simulink model "MX_Gateway"  (Simulink 9.0)
settings: Solver=FixedStepDiscrete  StartTime=0.0  StopTime=100  FixedStep=0.01  RelTol=1e-3
values from neighbouring files (symbols this model uses):
  ACTIVE = Bobcat.Parameter; CreateDefine( ACTIVE                         ,1                          ,'Define' ,'defines.h' )    [defines.m]
  ACTIVE_MODE_ONE = Bobcat.Parameter; CreateDefine( ACTIVE_MODE_ONE                ,19                         ,'Define' ,'defines.h' )    [defines.m]
  AUTHENTICATED = Bobcat.Parameter; CreateDefine( AUTHENTICATED                  ,1                          ,'Define' ,'defines.h' )    [defines.m]
  AUX_EMEA_CONTROLS = Bobcat.Parameter; CreateDefine( AUX_EMEA_CONTROLS  ,1   ,'Define' ,'defines.h' )    [enums.m]
  AUX_FLOW_HIGH = Bobcat.Parameter; CreateDefine( AUX_FLOW_HIGH           ,4  ,'Define' ,'defines.h' )    [enums.m]
  AUX_FLOW_INACTIVE = Bobcat.Parameter; CreateDefine( AUX_FLOW_INACTIVE       ,0  ,'Define' ,'defines.h' )    [enums.m]
  AUX_FLOW_LOW = Bobcat.Parameter; CreateDefine( AUX_FLOW_LOW            ,1  ,'Define' ,'defines.h' )    [enums.m]
  AUX_FLOW_MEDIUM = Bobcat.Parameter; CreateDefine( AUX_FLOW_MEDIUM         ,2  ,'Define' ,'defines.h' )    [enums.m]
  AUX_FLOW_STANDARD = Bobcat.Parameter; CreateDefine( AUX_FLOW_STANDARD       ,3  ,'Define' ,'defines.h' )    [enums.m]
  AUX_NON_FUNCTIONAL = Bobcat.Parameter; CreateDefine( AUX_NON_FUNCTIONAL ,255 ,'Define' ,'defines.h' )    [enums.m]
  BASE_ACTIVE = Bobcat.Parameter; CreateDefine( BASE_ACTIVE                    ,253                        ,'Define' ,'defines.h' )    [defines.m]
  BASE_DETENT = Bobcat.Parameter; CreateDefine( BASE_DETENT                    ,1                          ,'Define' ,'defines.h' )    [defines.m]
  BEEP_NONE = Bobcat.Parameter; CreateDefine( BEEP_NONE                      ,255                        ,'Define' ,'defines.h' )    [defines.m]
  CD_AIR_FILTER_DEBOUNCE_NORMAL = Bobcat.Parameter; CreateAliasCD( CD_AIR_FILTER_DEBOUNCE_NORMAL                        ,uint16(500)                ,'ConstantDataPointer' ,'CONSTANT_DATA' ,'AIR_FILTER_DEBOUNCE_NORMAL')    [cdata.m]    (also in hc12_cdata.m; m4_cdata.m)
  CD_AIR_FILTER_DEBOUNCE_NOT_CONNECTED = Bobcat.Parameter; CreateAliasCD( CD_AIR_FILTER_DEBOUNCE_NOT_CONNECTED                 ,uint16(3000)               ,'ConstantDataPointer' ,'CONSTANT_DATA' ,'AIR_FILTER_DEBOUNCE_NOT_CONNECTED')    [cdata.m]    (also in hc12_cdata.m; m4_cdata.m)
  CD_AIR_FILTER_DEBOUNCE_PLUGGED = Bobcat.Parameter; CreateAliasCD( CD_AIR_FILTER_DEBOUNCE_PLUGGED                       ,uint16(3000)               ,'ConstantDataPointer' ,'CONSTANT_DATA' ,'AIR_FILTER_DEBOUNCE_PLUGGED')    [cdata.m]    (also in hc12_cdata.m; m4_cdata.m)
  CD_AIR_FILTER_FUNCTION = Bobcat.Parameter; CreateAliasCD( CD_AIR_FILTER_FUNCTION                               ,uint8(255)                 ,'ConstantDataPointer' ,'CONSTANT_DATA' ,'AIR_FILTER_FUNCTION')    [cdata.m]    (also in hc12_cdata.m; m4_cdata.m)
  CD_AIR_FILTER_PLUGGED_WEIGHT = Bobcat.Parameter; CreateAliasCD( CD_AIR_FILTER_PLUGGED_WEIGHT                         ,uint8(2)                   ,'ConstantDataPointer' ,'CONSTANT_DATA' ,'AIR_FILTER_PLUGGED_WEIGHT')    [cdata.m]    (also in hc12_cdata.m; m4_cdata.m)
  CD_AIR_FILTER_UNPLUGGED_WEIGHT = Bobcat.Parameter; CreateAliasCD( CD_AIR_FILTER_UNPLUGGED_WEIGHT                       ,uint8(1)                   ,'ConstantDataPointer' ,'CONSTANT_DATA' ,'AIR_FILTER_UNPLUGGED_WEIGHT')    [cdata.m]    (also in hc12_cdata.m; m4_cdata.m)
  CD_AUTOIDLE_ARMBAR_DELAY = Bobcat.Parameter; CreateAliasCD( CD_AUTOIDLE_ARMBAR_DELAY                             ,uint8(2)                   ,'ConstantDataPointer' ,'CONSTANT_DATA' ,'AUTOIDLE_ARMBAR_DELAY')    [cdata.m]    (also in hc12_cdata.m; m4_cdata.m)
  CD_AUTOIDLE_FUNCTION = Bobcat.Parameter; CreateAliasCD( CD_AUTOIDLE_FUNCTION                                 ,uint8(255)                 ,'ConstantDataPointer' ,'CONSTANT_DATA' ,'AUTOIDLE_FUNCTION')    [cdata.m]    (also in hc12_cdata.m; m4_cdata.m)
  CD_AUTOIDLE_IDLE_DELAY = Bobcat.Parameter; CreateAliasCD( CD_AUTOIDLE_IDLE_DELAY                               ,uint8(40)                  ,'ConstantDataPointer' ,'CONSTANT_DATA' ,'AUTOIDLE_IDLE_DELAY')    [cdata.m]    (also in hc12_cdata.m; m4_cdata.m)
  CD_AUTOIDLE_LOW_IDLE_START = Bobcat.Parameter; CreateAliasCD( CD_AUTOIDLE_LOW_IDLE_START                           ,uint8(255)                 ,'ConstantDataPointer' ,'CONSTANT_DATA' ,'AUTOIDLE_LOW_IDLE_START')    [cdata.m]    (also in hc12_cdata.m; m4_cdata.m)
  CD_AUTOIDLE_PRESSURE_OFF = Bobcat.Parameter; CreateAliasCD( CD_AUTOIDLE_PRESSURE_OFF                             ,uint16(300)                ,'ConstantDataPointer' ,'CONSTANT_DATA' ,'AUTOIDLE_PRESSURE_OFF')    [cdata.m]    (also in hc12_cdata.m; m4_cdata.m)
  CD_AUTOIDLE_PRESSURE_ON = Bobcat.Parameter; CreateAliasCD( CD_AUTOIDLE_PRESSURE_ON                              ,uint16(270)                ,'ConstantDataPointer' ,'CONSTANT_DATA' ,'AUTOIDLE_PRESSURE_ON')    [cdata.m]    (also in hc12_cdata.m; m4_cdata.m)
  CD_AUTOSHIFT_FUNCTION = Bobcat.Parameter; CreateAliasCD( CD_AUTOSHIFT_FUNCTION                                ,uint8(255)                 ,'ConstantDataPointer' ,'CONSTANT_DATA' ,'AUTOSHIFT_FUNCTION')    [cdata.m]    (also in hc12_cdata.m; m4_cdata.m)
  CD_AUTOSHIFT_PHIGH1 = Bobcat.Parameter; CreateAliasCD( CD_AUTOSHIFT_PHIGH1                                  ,uint16(205)                ,'ConstantDataPointer' ,'CONSTANT_DATA' ,'AUTOSHIFT_PHIGH1')    [cdata.m]    (also in hc12_cdata.m; m4_cdata.m)
  CD_AUTOSHIFT_PHIGH2 = Bobcat.Parameter; CreateAliasCD( CD_AUTOSHIFT_PHIGH2                                  ,uint16(193)                ,'ConstantDataPointer' ,'CONSTANT_DATA' ,'AUTOSHIFT_PHIGH2')    [cdata.m]    (also in hc12_cdata.m; m4_cdata.m)
  CD_AUTOSHIFT_PHIGH_TEMP1 = Bobcat.Parameter; CreateAliasCD( CD_AUTOSHIFT_PHIGH_TEMP1                             ,int16(70)                  ,'ConstantDataPointer' ,'CONSTANT_DATA' ,'AUTOSHIFT_PHIGH_TEMP1')    [cdata.m]    (also in hc12_cdata.m; m4_cdata.m)
  CD_AUTOSHIFT_PHIGH_TEMP2 = Bobcat.Parameter; CreateAliasCD( CD_AUTOSHIFT_PHIGH_TEMP2                             ,int16(160)                 ,'ConstantDataPointer' ,'CONSTANT_DATA' ,'AUTOSHIFT_PHIGH_TEMP2')    [cdata.m]    (also in hc12_cdata.m; m4_cdata.m)
  CD_AUTOSHIFT_PLOW1 = Bobcat.Parameter; CreateAliasCD( CD_AUTOSHIFT_PLOW1                                   ,uint16(130)                ,'ConstantDataPointer' ,'CONSTANT_DATA' ,'AUTOSHIFT_PLOW1')    [cdata.m]    (also in hc12_cdata.m; m4_cdata.m)
  CD_AUTOSHIFT_PLOW2 = Bobcat.Parameter; CreateAliasCD( CD_AUTOSHIFT_PLOW2                                   ,uint16(100)                ,'ConstantDataPointer' ,'CONSTANT_DATA' ,'AUTOSHIFT_PLOW2')    [cdata.m]    (also in hc12_cdata.m; m4_cdata.m)
  CD_AUTOSHIFT_PLOW_TEMP1 = Bobcat.Parameter; CreateAliasCD( CD_AUTOSHIFT_PLOW_TEMP1                              ,int16(20)                  ,'ConstantDataPointer' ,'CONSTANT_DATA' ,'AUTOSHIFT_PLOW_TEMP1')    [cdata.m]    (also in hc12_cdata.m; m4_cdata.m)
  CD_AUTOSHIFT_PLOW_TEMP2 = Bobcat.Parameter; CreateAliasCD( CD_AUTOSHIFT_PLOW_TEMP2                              ,int16(80)                  ,'ConstantDataPointer' ,'CONSTANT_DATA' ,'AUTOSHIFT_PLOW_TEMP2')    [cdata.m]    (also in hc12_cdata.m; m4_cdata.m)
  CD_AUTOSHIFT_TRAVEL_DELAY = Bobcat.Parameter; CreateAliasCD( CD_AUTOSHIFT_TRAVEL_DELAY                            ,uint8(32)                  ,'ConstantDataPointer' ,'CONSTANT_DATA' ,'AUTOSHIFT_TRAVEL_DELAY')    [cdata.m]    (also in hc12_cdata.m; m4_cdata.m)
  CD_AUX2_CRACKPOINT_CURRENT = Bobcat.Parameter; CreateAliasCD( CD_AUX2_CRACKPOINT_CURRENT                           ,fi(0,0,16,12,'hex','0c28') ,'ConstantDataPointer' ,'CONSTANT_DATA' ,'AUX2_CRACKPOINT_CURRENT')    [cdata.m]    (also in hc12_cdata.m; m4_cdata.m)
  CD_AUX2_FLOW_LOW_FUNCTION = Bobcat.Parameter; CreateAliasCD( CD_AUX2_FLOW_LOW_FUNCTION                            ,uint8(255)                 ,'ConstantDataPointer' ,'CONSTANT_DATA' ,'AUX2_FLOW_LOW_FUNCTION')    [cdata.m]    (also in hc12_cdata.m; m4_cdata.m)
  CD_AUX2_FLOW_MED_FUNCTION = Bobcat.Parameter; CreateAliasCD( CD_AUX2_FLOW_MED_FUNCTION                            ,uint8(255)                 ,'ConstantDataPointer' ,'CONSTANT_DATA' ,'AUX2_FLOW_MED_FUNCTION')    [cdata.m]    (also in hc12_cdata.m; m4_cdata.m)
  CD_AUX2_HI_FLOW_PERCENT = Bobcat.Parameter; CreateAliasCD( CD_AUX2_HI_FLOW_PERCENT                              ,fi(0,0,16,16,'hex','ffff') ,'ConstantDataPointer' ,'CONSTANT_DATA' ,'AUX2_HI_FLOW_PERCENT')    [cdata.m]    (also in hc12_cdata.m; m4_cdata.m)
  CD_AUX2_LOW_FLOW_PERCENT = Bobcat.Parameter; CreateAliasCD( CD_AUX2_LOW_FLOW_PERCENT                             ,fi(0,0,16,16,'hex','7333') ,'ConstantDataPointer' ,'CONSTANT_DATA' ,'AUX2_LOW_FLOW_PERCENT')    [cdata.m]    (also in hc12_cdata.m; m4_cdata.m)
  CD_AUX2_MAX_FLOW_CURRENT = Bobcat.Parameter; CreateAliasCD( CD_AUX2_MAX_FLOW_CURRENT                             ,fi(0,0,16,12,'hex','14cc') ,'ConstantDataPointer' ,'CONSTANT_DATA' ,'AUX2_MAX_FLOW_CURRENT')    [cdata.m]    (also in hc12_cdata.m; m4_cdata.m)
  CD_AUX2_MED_FLOW_PERCENT = Bobcat.Parameter; CreateAliasCD( CD_AUX2_MED_FLOW_PERCENT                             ,fi(0,0,16,16,'hex','9999') ,'ConstantDataPointer' ,'CONSTANT_DATA' ,'AUX2_MED_FLOW_PERCENT')    [cdata.m]    (also in hc12_cdata.m; m4_cdata.m)
  CD_AUX_CRACKPOINT_CURRENT = Bobcat.Parameter; CreateAliasCD( CD_AUX_CRACKPOINT_CURRENT                            ,fi(0,0,16,12,'hex','0000') ,'ConstantDataPointer' ,'CONSTANT_DATA' ,'AUX_CRACKPOINT_CURRENT')    [cdata.m]    (also in hc12_cdata.m; m4_cdata.m)
  CD_AUX_DETENT_FLASH_TIME = Bobcat.Parameter; CreateAliasCD( CD_AUX_DETENT_FLASH_TIME                             ,uint16(72)                 ,'ConstantDataPointer' ,'CONSTANT_DATA' ,'AUX_DETENT_FLASH_TIME')    [cdata.m]    (also in hc12_cdata.m; m4_cdata.m)
  CD_AUX_DETENT_HOLD_TIME = Bobcat.Parameter; CreateAliasCD( CD_AUX_DETENT_HOLD_TIME                              ,uint16(100)                ,'ConstantDataPointer' ,'CONSTANT_DATA' ,'AUX_DETENT_HOLD_TIME')    [cdata.m]    (also in hc12_cdata.m; m4_cdata.m)
  CD_AUX_FULL_ON_AUX1 = Bobcat.Parameter; CreateAliasCD( CD_AUX_FULL_ON_AUX1                                  ,fi(0,0,16,12,'hex','10cd') ,'ConstantDataPointer' ,'CONSTANT_DATA' ,'AUX_FULL_ON_AUX1')    [cdata.m]    (also in hc12_cdata.m; m4_cdata.m)
  CD_AUX_FULL_ON_AUX2 = Bobcat.Parameter; CreateAliasCD( CD_AUX_FULL_ON_AUX2                                  ,fi(0,0,16,12,'hex','139d') ,'ConstantDataPointer' ,'CONSTANT_DATA' ,'AUX_FULL_ON_AUX2')    [cdata.m]    (also in hc12_cdata.m; m4_cdata.m)
  CD_AUX_FULL_ON_AUX3 = Bobcat.Parameter; CreateAliasCD( CD_AUX_FULL_ON_AUX3                                  ,fi(0,0,16,12,'hex','17d7') ,'ConstantDataPointer' ,'CONSTANT_DATA' ,'AUX_FULL_ON_AUX3')    [cdata.m]    (also in hc12_cdata.m; m4_cdata.m)
  CD_AUX_FUNCTION = Bobcat.Parameter; CreateAliasCD( CD_AUX_FUNCTION                                      ,uint8(0)                   ,'ConstantDataPointer' ,'CONSTANT_DATA' ,'AUX_FUNCTION')    [cdata.m]    (also in hc12_cdata.m; m4_cdata.m)
  CD_AUX_RATE_LIMIT_CRACKPOINT = Bobcat.Parameter; CreateAliasCD( CD_AUX_RATE_LIMIT_CRACKPOINT                         ,fi(0,0,16,12,'hex','0800') ,'ConstantDataPointer' ,'CONSTANT_DATA' ,'AUX_RATE_LIMIT_CRACKPOINT')    [cdata.m]    (also in hc12_cdata.m; m4_cdata.m)
  CD_AUX_RATE_LIMIT_CRACKPOINT_RATE = Bobcat.Parameter; CreateAliasCD( CD_AUX_RATE_LIMIT_CRACKPOINT_RATE                    ,fi(0,1,32,12,'int',102400) ,'ConstantDataPointer' ,'CONSTANT_DATA' ,'AUX_RATE_LIMIT_CRACKPOINT_RATE')    [cdata.m]    (also in hc12_cdata.m; m4_cdata.m)
  CD_AUX_RATE_LIMIT_FUNCTION = Bobcat.Parameter; CreateAliasCD( CD_AUX_RATE_LIMIT_FUNCTION                           ,uint8(255)                 ,'ConstantDataPointer' ,'CONSTANT_DATA' ,'AUX_RATE_LIMIT_FUNCTION')    [cdata.m]    (also in hc12_cdata.m; m4_cdata.m)
  CD_AUX_RATE_LIMIT_MAX_ENG_SPD = Bobcat.Parameter; CreateAliasCD( CD_AUX_RATE_LIMIT_MAX_ENG_SPD                        ,uint16(1600)               ,'ConstantDataPointer' ,'CONSTANT_DATA' ,'AUX_RATE_LIMIT_MAX_ENG_SPD')    [cdata.m]    (also in hc12_cdata.m; m4_cdata.m)
  CD_AUX_RATE_LIMIT_MIN_ENG_SPD = Bobcat.Parameter; CreateAliasCD( CD_AUX_RATE_LIMIT_MIN_ENG_SPD                        ,uint16(100)                ,'ConstantDataPointer' ,'CONSTANT_DATA' ,'AUX_RATE_LIMIT_MIN_ENG_SPD')    [cdata.m]    (also in hc12_cdata.m; m4_cdata.m)
  CD_AUX_RATE_LIMIT_NORMAL_RATE = Bobcat.Parameter; CreateAliasCD( CD_AUX_RATE_LIMIT_NORMAL_RATE                        ,fi(0,1,32,12,'int',2048)   ,'ConstantDataPointer' ,'CONSTANT_DATA' ,'AUX_RATE_LIMIT_NORMAL_RATE')    [cdata.m]    (also in hc12_cdata.m; m4_cdata.m)
  CD_AUXPOT_CAL_DEADBAND_HIGH = Bobcat.Parameter; CreateAliasCD( CD_AUXPOT_CAL_DEADBAND_HIGH                          ,uint16(574)                ,'ConstantDataPointer' ,'CONSTANT_DATA' ,'AUXPOT_CAL_DEADBAND_HIGH')    [cdata.m]    (also in hc12_cdata.m; m4_cdata.m)
  CD_AUXPOT_CAL_DEADBAND_LOW = Bobcat.Parameter; CreateAliasCD( CD_AUXPOT_CAL_DEADBAND_LOW                           ,uint16(424)                ,'ConstantDataPointer' ,'CONSTANT_DATA' ,'AUXPOT_CAL_DEADBAND_LOW')    [cdata.m]    (also in hc12_cdata.m; m4_cdata.m)
  CD_AUXPOT_CENTER_RATIO = Bobcat.Parameter; CreateAliasCD( CD_AUXPOT_CENTER_RATIO                               ,uint16(500)                ,'ConstantDataPointer' ,'CONSTANT_DATA' ,'AUXPOT_CENTER_RATIO')    [cdata.m]    (also in hc12_cdata.m; m4_cdata.m)
  CD_AUXPOT_DEADBAND = Bobcat.Parameter; CreateAliasCD( CD_AUXPOT_DEADBAND                                   ,uint8(38)                  ,'ConstantDataPointer' ,'CONSTANT_DATA' ,'AUXPOT_DEADBAND')    [cdata.m]    (also in hc12_cdata.m; m4_cdata.m)
  CD_AUXPOT_FUNCTION = Bobcat.Parameter; CreateAliasCD( CD_AUXPOT_FUNCTION                                   ,uint8(0)                   ,'ConstantDataPointer' ,'CONSTANT_DATA' ,'AUXPOT_FUNCTION')    [cdata.m]    (also in hc12_cdata.m; m4_cdata.m)
  CD_AUXPOT_RATIO_GAIN = Bobcat.Parameter; CreateAliasCD( CD_AUXPOT_RATIO_GAIN                                 ,uint16(1000)               ,'ConstantDataPointer' ,'CONSTANT_DATA' ,'AUXPOT_RATIO_GAIN')    [cdata.m]    (also in hc12_cdata.m; m4_cdata.m)
  CD_CAN_THROTTLE_FUNCTION = Bobcat.Parameter; CreateAliasCD( CD_CAN_THROTTLE_FUNCTION                             ,uint8(255)                 ,'ConstantDataPointer' ,'CONSTANT_DATA' ,'CAN_THROTTLE_FUNCTION')    [cdata.m]    (also in hc12_cdata.m; m4_cdata.m)
  CD_CAN_THROTTLE_REVERSE = Bobcat.Parameter; CreateAliasCD( CD_CAN_THROTTLE_REVERSE                              ,uint8(255)                 ,'ConstantDataPointer' ,'CONSTANT_DATA' ,'CAN_THROTTLE_REVERSE')    [cdata.m]    (also in hc12_cdata.m; m4_cdata.m)
  CD_CONSOLE_DEBOUNCE_ABSENT = Bobcat.Parameter; CreateAliasCD( CD_CONSOLE_DEBOUNCE_ABSENT                           ,uint16(12)                 ,'ConstantDataPointer' ,'CONSTANT_DATA' ,'CONSOLE_DEBOUNCE_ABSENT')    [cdata.m]    (also in hc12_cdata.m; m4_cdata.m)
  CD_CONSOLE_DEBOUNCE_OORH = Bobcat.Parameter; CreateAliasCD( CD_CONSOLE_DEBOUNCE_OORH                             ,uint16(12)                 ,'ConstantDataPointer' ,'CONSTANT_DATA' ,'CONSOLE_DEBOUNCE_OORH')    [cdata.m]    (also in hc12_cdata.m; m4_cdata.m)
  CD_CONSOLE_DEBOUNCE_OORL = Bobcat.Parameter; CreateAliasCD( CD_CONSOLE_DEBOUNCE_OORL                             ,uint16(12)                 ,'ConstantDataPointer' ,'CONSTANT_DATA' ,'CONSOLE_DEBOUNCE_OORL')    [cdata.m]    (also in hc12_cdata.m; m4_cdata.m)
  CD_CONSOLE_DEBOUNCE_PRESENT = Bobcat.Parameter; CreateAliasCD( CD_CONSOLE_DEBOUNCE_PRESENT                          ,uint16(12)                 ,'ConstantDataPointer' ,'CONSTANT_DATA' ,'CONSOLE_DEBOUNCE_PRESENT')    [cdata.m]    (also in hc12_cdata.m; m4_cdata.m)
  CD_CONSOLE_FUNCTION = Bobcat.Parameter; CreateAliasCD( CD_CONSOLE_FUNCTION                                  ,uint8(0)                   ,'ConstantDataPointer' ,'CONSTANT_DATA' ,'CONSOLE_FUNCTION')    [cdata.m]    (also in hc12_cdata.m; m4_cdata.m)
  CD_CONSOLE_OORH = Bobcat.Parameter; CreateAliasCD( CD_CONSOLE_OORH                                      ,uint16(740)                ,'ConstantDataPointer' ,'CONSTANT_DATA' ,'CONSOLE_OORH')    [cdata.m]    (also in hc12_cdata.m; m4_cdata.m)
  CD_CONSOLE_OORL = Bobcat.Parameter; CreateAliasCD( CD_CONSOLE_OORL                                      ,uint16(10)                 ,'ConstantDataPointer' ,'CONSTANT_DATA' ,'CONSOLE_OORL')    [cdata.m]    (also in hc12_cdata.m; m4_cdata.m)
  CD_CONSOLE_PRESENT_HIGH = Bobcat.Parameter; CreateAliasCD( CD_CONSOLE_PRESENT_HIGH                              ,uint16(515)                ,'ConstantDataPointer' ,'CONSTANT_DATA' ,'CONSOLE_PRESENT_HIGH')    [cdata.m]    (also in hc12_cdata.m; m4_cdata.m)
  CD_CONSOLE_PRESENT_LOW = Bobcat.Parameter; CreateAliasCD( CD_CONSOLE_PRESENT_LOW                               ,uint16(375)                ,'ConstantDataPointer' ,'CONSTANT_DATA' ,'CONSOLE_PRESENT_LOW')    [cdata.m]    (also in hc12_cdata.m; m4_cdata.m)
  CD_DELUXE_GEN5_5_FUNCTION = Bobcat.Parameter; CreateAliasCD( CD_DELUXE_GEN5_5_FUNCTION                            ,uint8(255)                 ,'ConstantDataPointer' ,'CONSTANT_DATA' ,'DELUXE_GEN5_5_FUNCTION')    [cdata.m]    (also in hc12_cdata.m; m4_cdata.m)
  CD_DELUXE_GEN5_FUNCTION = Bobcat.Parameter; CreateAliasCD( CD_DELUXE_GEN5_FUNCTION                              ,uint8(255)                 ,'ConstantDataPointer' ,'CONSTANT_DATA' ,'DELUXE_GEN5_FUNCTION')    [cdata.m]    (also in hc12_cdata.m; m4_cdata.m)
  CD_DERATE_ENGINE_SPEED_DEBOUNCE_HIGH = Bobcat.Parameter; CreateAliasCD( CD_DERATE_ENGINE_SPEED_DEBOUNCE_HIGH                 ,uint16(200)                ,'ConstantDataPointer' ,'CONSTANT_DATA' ,'DERATE_ENGINE_SPEED_DEBOUNCE_HIGH')    [cdata.m]    (also in hc12_cdata.m; m4_cdata.m)
  CD_DERATE_ENGINE_SPEED_DEBOUNCE_SHUTDOWN = Bobcat.Parameter; CreateAliasCD( CD_DERATE_ENGINE_SPEED_DEBOUNCE_SHUTDOWN             ,uint16(1000)               ,'ConstantDataPointer' ,'CONSTANT_DATA' ,'DERATE_ENGINE_SPEED_DEBOUNCE_SHUTDOWN')    [cdata.m]    (also in hc12_cdata.m; m4_cdata.m)
  CD_DERATE_ENGINE_SPEED_HIGH_OFFSET = Bobcat.Parameter; CreateAliasCD( CD_DERATE_ENGINE_SPEED_HIGH_OFFSET                   ,uint16(200)                ,'ConstantDataPointer' ,'CONSTANT_DATA' ,'DERATE_ENGINE_SPEED_HIGH_OFFSET')    [cdata.m]    (also in hc12_cdata.m; m4_cdata.m)
  CD_DERATE_METHOD = Bobcat.Parameter; CreateAliasCD( CD_DERATE_METHOD                                     ,uint8(0)                   ,'ConstantDataPointer' ,'CONSTANT_DATA' ,'DERATE_METHOD')    [cdata.m]    (also in hc12_cdata.m; m4_cdata.m)
  CD_DUMP_VALVE_FUNCTION = Bobcat.Parameter; CreateAliasCD( CD_DUMP_VALVE_FUNCTION                               ,uint8(255)                 ,'ConstantDataPointer' ,'CONSTANT_DATA' ,'DUMP_VALVE_FUNCTION')    [cdata.m]    (also in hc12_cdata.m; m4_cdata.m)
  CD_DUMP_VALVE_ON_CURRENT = Bobcat.Parameter; CreateAliasCD( CD_DUMP_VALVE_ON_CURRENT                             ,fi(0,0,16,12,'hex','ffff') ,'ConstantDataPointer' ,'CONSTANT_DATA' ,'DUMP_VALVE_ON_CURRENT')    [cdata.m]    (also in hc12_cdata.m; m4_cdata.m)
  CD_ECO_MODE_THROTTLE_REDUCTION = Bobcat.Parameter; CreateAliasCD( CD_ECO_MODE_THROTTLE_REDUCTION                       ,uint16(850)                ,'ConstantDataPointer' ,'CONSTANT_DATA' ,'ECO_MODE_THROTTLE_REDUCTION')    [cdata.m]    (also in hc12_cdata.m; m4_cdata.m)
  CD_ECT_FILTER_GAIN = Bobcat.Parameter; CreateAliasCD( CD_ECT_FILTER_GAIN                                   ,uint8(6)                   ,'ConstantDataPointer' ,'CONSTANT_DATA' ,'ECT_FILTER_GAIN')    [cdata.m]    (also in hc12_cdata.m; m4_cdata.m)
  CD_ECU_FUNCTION = Bobcat.Parameter; CreateAliasCD( CD_ECU_FUNCTION                                      ,uint8(255)                 ,'ConstantDataPointer' ,'CONSTANT_DATA' ,'ECU_FUNCTION')    [cdata.m]    (also in hc12_cdata.m; m4_cdata.m)
  CD_ECU_HOLD_DEBOUNCE_ERROR_ON = Bobcat.Parameter; CreateAliasCD( CD_ECU_HOLD_DEBOUNCE_ERROR_ON                        ,uint16(20)                 ,'ConstantDataPointer' ,'CONSTANT_DATA' ,'ECU_HOLD_DEBOUNCE_ERROR_ON')    [cdata.m]    (also in hc12_cdata.m; m4_cdata.m)
  CD_EMEA_CONTROLS_HOLD_TIME = Bobcat.Parameter; CreateAliasCD( CD_EMEA_CONTROLS_HOLD_TIME                           ,uint16(30)                 ,'ConstantDataPointer' ,'CONSTANT_DATA' ,'EMEA_CONTROLS_HOLD_TIME')    [cdata.m]    (also in hc12_cdata.m; m4_cdata.m)
  CD_EMEA_CONTROLS_OPTION_A = Bobcat.Parameter; CreateAliasCD( CD_EMEA_CONTROLS_OPTION_A                            ,uint8(255)                 ,'ConstantDataPointer' ,'CONSTANT_DATA' ,'EMEA_CONTROLS_OPTION_A')    [cdata.m]    (also in hc12_cdata.m; m4_cdata.m)
  CD_EMEA_CONTROLS_OPTION_C = Bobcat.Parameter; CreateAliasCD( CD_EMEA_CONTROLS_OPTION_C                            ,uint8(255)                 ,'ConstantDataPointer' ,'CONSTANT_DATA' ,'EMEA_CONTROLS_OPTION_C')    [cdata.m]    (also in hc12_cdata.m; m4_cdata.m)
  CD_EMEA_CONTROLS_REVERSE = Bobcat.Parameter; CreateAliasCD( CD_EMEA_CONTROLS_REVERSE                             ,uint8(255)                 ,'ConstantDataPointer' ,'CONSTANT_DATA' ,'EMEA_CONTROLS_REVERSE')    [cdata.m]    (also in hc12_cdata.m; m4_cdata.m)
  CD_EMEA_ELECTRIC_VERSION = Bobcat.Parameter; CreateAliasCD( CD_EMEA_ELECTRIC_VERSION                             ,uint8(0)                   ,'ConstantDataPointer' ,'CONSTANT_DATA' ,'EMEA_ELECTRIC_VERSION')    [cdata.m]    (also in hc12_cdata.m; m4_cdata.m)
  CD_EMEA_POT_CAL_DEADBAND_HIGH = Bobcat.Parameter; CreateAliasCD( CD_EMEA_POT_CAL_DEADBAND_HIGH                        ,uint16(574)                ,'ConstantDataPointer' ,'CONSTANT_DATA' ,'EMEA_POT_CAL_DEADBAND_HIGH')    [cdata.m]    (also in hc12_cdata.m; m4_cdata.m)
  CD_EMEA_POT_CAL_DEADBAND_LOW = Bobcat.Parameter; CreateAliasCD( CD_EMEA_POT_CAL_DEADBAND_LOW                         ,uint16(424)                ,'ConstantDataPointer' ,'CONSTANT_DATA' ,'EMEA_POT_CAL_DEADBAND_LOW')    [cdata.m]    (also in hc12_cdata.m; m4_cdata.m)
  CD_ENGINE_RPM_FACTOR = Bobcat.Parameter; CreateAliasCD( CD_ENGINE_RPM_FACTOR                                 ,uint8(35)                  ,'ConstantDataPointer' ,'CONSTANT_DATA' ,'ENGINE_RPM_FACTOR')    [cdata.m]    (also in hc12_cdata.m; m4_cdata.m)
  CD_ENGINE_SPEED_FILTER_FAST_VALUE = Bobcat.Parameter; CreateAliasCD( CD_ENGINE_SPEED_FILTER_FAST_VALUE                    ,uint8(1)                   ,'ConstantDataPointer' ,'CONSTANT_DATA' ,'ENGINE_SPEED_FILTER_FAST_VALUE')    [cdata.m]    (also in hc12_cdata.m; m4_cdata.m)
  CD_ENGINE_SPEED_FILTER_SLOW_VALUE = Bobcat.Parameter; CreateAliasCD( CD_ENGINE_SPEED_FILTER_SLOW_VALUE                    ,uint8(4)                   ,'ConstantDataPointer' ,'CONSTANT_DATA' ,'ENGINE_SPEED_FILTER_SLOW_VALUE')    [cdata.m]    (also in hc12_cdata.m; m4_cdata.m)
  CD_ENGINE_SPEED_RUNNING = Bobcat.Parameter; CreateAliasCD( CD_ENGINE_SPEED_RUNNING                              ,uint16(800)                ,'ConstantDataPointer' ,'CONSTANT_DATA' ,'ENGINE_SPEED_RUNNING')    [cdata.m]    (also in hc12_cdata.m; m4_cdata.m)
  CD_ENGINE_START_DELAY_DEBOUNCE_RPM_NO_SIGNAL = Bobcat.Parameter; CreateAliasCD( CD_ENGINE_START_DELAY_DEBOUNCE_RPM_NO_SIGNAL         ,uint16(0)                  ,'ConstantDataPointer' ,'CONSTANT_DATA' ,'ENGINE_START_DELAY_DEBOUNCE_RPM_NO_SIGNAL')    [cdata.m]    (also in hc12_cdata.m; m4_cdata.m)
  CD_ENGINE_START_DELAY_OFF_TIME = Bobcat.Parameter; CreateAliasCD( CD_ENGINE_START_DELAY_OFF_TIME                       ,uint16(0)                  ,'ConstantDataPointer' ,'CONSTANT_DATA' ,'ENGINE_START_DELAY_OFF_TIME')    [cdata.m]    (also in hc12_cdata.m; m4_cdata.m)
  CD_ENGINE_START_DELAY_RPM_NO_SIGNAL = Bobcat.Parameter; CreateAliasCD( CD_ENGINE_START_DELAY_RPM_NO_SIGNAL                  ,uint16(0)                  ,'ConstantDataPointer' ,'CONSTANT_DATA' ,'ENGINE_START_DELAY_RPM_NO_SIGNAL')    [cdata.m]    (also in hc12_cdata.m; m4_cdata.m)
  CD_EOP_DEBOUNCE_EXTREMELY_LOW = Bobcat.Parameter; CreateAliasCD( CD_EOP_DEBOUNCE_EXTREMELY_LOW                        ,uint16(50)                 ,'ConstantDataPointer' ,'CONSTANT_DATA' ,'EOP_DEBOUNCE_EXTREMELY_LOW')    [cdata.m]    (also in hc12_cdata.m; m4_cdata.m)
  CD_EOP_DEBOUNCE_NORMAL = Bobcat.Parameter; CreateAliasCD( CD_EOP_DEBOUNCE_NORMAL                               ,uint16(50)                 ,'ConstantDataPointer' ,'CONSTANT_DATA' ,'EOP_DEBOUNCE_NORMAL')    [cdata.m]    (also in hc12_cdata.m; m4_cdata.m)
  CD_EOP_DEBOUNCE_SHUTDOWN = Bobcat.Parameter; CreateAliasCD( CD_EOP_DEBOUNCE_SHUTDOWN                             ,uint16(100)                ,'ConstantDataPointer' ,'CONSTANT_DATA' ,'EOP_DEBOUNCE_SHUTDOWN')    [cdata.m]    (also in hc12_cdata.m; m4_cdata.m)
  CD_EOP_FILTER_VALUE = Bobcat.Parameter; CreateAliasCD( CD_EOP_FILTER_VALUE                                  ,uint8(3)                   ,'ConstantDataPointer' ,'CONSTANT_DATA' ,'EOP_FILTER_VALUE')    [cdata.m]    (also in hc12_cdata.m; m4_cdata.m)
  CD_EOP_FUNCTION = Bobcat.Parameter; CreateAliasCD( CD_EOP_FUNCTION                                      ,uint8(1)                   ,'ConstantDataPointer' ,'CONSTANT_DATA' ,'EOP_FUNCTION')    [cdata.m]    (also in hc12_cdata.m; m4_cdata.m)
  CD_EOP_SWITCH_GAUGE_VALUE = Bobcat.Parameter; CreateAliasCD( CD_EOP_SWITCH_GAUGE_VALUE                            ,uint16(125)                ,'ConstantDataPointer' ,'CONSTANT_DATA' ,'EOP_SWITCH_GAUGE_VALUE')    [cdata.m]    (also in hc12_cdata.m; m4_cdata.m)
  CD_EOP_SWITCH_LOW = Bobcat.Parameter; CreateAliasCD( CD_EOP_SWITCH_LOW                                    ,uint16(50)                 ,'ConstantDataPointer' ,'CONSTANT_DATA' ,'EOP_SWITCH_LOW')    [cdata.m]    (also in hc12_cdata.m; m4_cdata.m)
  CD_EOP_SWITCH_PRESSURE_VALUE = Bobcat.Parameter; CreateAliasCD( CD_EOP_SWITCH_PRESSURE_VALUE                         ,uint16(40)                 ,'ConstantDataPointer' ,'CONSTANT_DATA' ,'EOP_SWITCH_PRESSURE_VALUE')    [cdata.m]    (also in hc12_cdata.m; m4_cdata.m)
  CD_EOT_DEBOUNCE_EXTREMELY_LOW = Bobcat.Parameter; CreateAliasCD( CD_EOT_DEBOUNCE_EXTREMELY_LOW                        ,uint16(2)                  ,'ConstantDataPointer' ,'CONSTANT_DATA' ,'EOT_DEBOUNCE_EXTREMELY_LOW')    [cdata.m]    (also in hc12_cdata.m; m4_cdata.m)
  CD_EOT_DEBOUNCE_LOW = Bobcat.Parameter; CreateAliasCD( CD_EOT_DEBOUNCE_LOW                                  ,uint16(2)                  ,'ConstantDataPointer' ,'CONSTANT_DATA' ,'EOT_DEBOUNCE_LOW')    [cdata.m]    (also in hc12_cdata.m; m4_cdata.m)
  CD_EOT_DEBOUNCE_NORMAL = Bobcat.Parameter; CreateAliasCD( CD_EOT_DEBOUNCE_NORMAL                               ,uint16(2)                  ,'ConstantDataPointer' ,'CONSTANT_DATA' ,'EOT_DEBOUNCE_NORMAL')    [cdata.m]    (also in hc12_cdata.m; m4_cdata.m)
  CD_EOT_EXTREMELY_LOW = Bobcat.Parameter; CreateAliasCD( CD_EOT_EXTREMELY_LOW                                 ,uint16(81)                 ,'ConstantDataPointer' ,'CONSTANT_DATA' ,'EOT_EXTREMELY_LOW')    [cdata.m]    (also in hc12_cdata.m; m4_cdata.m)
  CD_EOT_FUNCTION = Bobcat.Parameter; CreateAliasCD( CD_EOT_FUNCTION                                      ,uint8(255)                 ,'ConstantDataPointer' ,'CONSTANT_DATA' ,'EOT_FUNCTION')    [cdata.m]    (also in hc12_cdata.m; m4_cdata.m)
  CD_EOT_LOW = Bobcat.Parameter; CreateAliasCD( CD_EOT_LOW                                           ,uint16(90)                 ,'ConstantDataPointer' ,'CONSTANT_DATA' ,'EOT_LOW')    [cdata.m]    (also in hc12_cdata.m; m4_cdata.m)
  CD_EOT_TEMPERATURE_HYSTERESIS = Bobcat.Parameter; CreateAliasCD( CD_EOT_TEMPERATURE_HYSTERESIS                        ,uint16(2)                  ,'ConstantDataPointer' ,'CONSTANT_DATA' ,'EOT_TEMPERATURE_HYSTERESIS')    [cdata.m]    (also in hc12_cdata.m; m4_cdata.m)
  CD_FAST_TRAVEL_FUNCTION = Bobcat.Parameter; CreateAliasCD( CD_FAST_TRAVEL_FUNCTION                              ,uint8(255)                 ,'ConstantDataPointer' ,'CONSTANT_DATA' ,'FAST_TRAVEL_FUNCTION')    [cdata.m]    (also in hc12_cdata.m; m4_cdata.m)
  CD_FAST_TRAVEL_THROTTLE_DELAY = Bobcat.Parameter; CreateAliasCD( CD_FAST_TRAVEL_THROTTLE_DELAY                        ,uint16(10)                 ,'ConstantDataPointer' ,'CONSTANT_DATA' ,'FAST_TRAVEL_THROTTLE_DELAY')    [cdata.m]    (also in hc12_cdata.m; m4_cdata.m)
  CD_FAST_TRAVEL_THROTTLE_LIMIT = Bobcat.Parameter; CreateAliasCD( CD_FAST_TRAVEL_THROTTLE_LIMIT                        ,uint16(1000)               ,'ConstantDataPointer' ,'CONSTANT_DATA' ,'FAST_TRAVEL_THROTTLE_LIMIT')    [cdata.m]    (also in hc12_cdata.m; m4_cdata.m)
  CD_FLYWHEEL_TEETH = Bobcat.Parameter; CreateAliasCD( CD_FLYWHEEL_TEETH                                    ,uint8(83)                  ,'ConstantDataPointer' ,'CONSTANT_DATA' ,'FLYWHEEL_TEETH')    [cdata.m]    (also in hc12_cdata.m; m4_cdata.m)
  CD_FUEL_LEVEL_FILTER_FAST_VALUE = Bobcat.Parameter; CreateAliasCD( CD_FUEL_LEVEL_FILTER_FAST_VALUE                      ,uint8(5)                   ,'ConstantDataPointer' ,'CONSTANT_DATA' ,'FUEL_LEVEL_FILTER_FAST_VALUE')    [cdata.m]    (also in hc12_cdata.m; m4_cdata.m)
  CD_FUEL_LEVEL_FILTER_SLOW_VALUE = Bobcat.Parameter; CreateAliasCD( CD_FUEL_LEVEL_FILTER_SLOW_VALUE                      ,uint8(10)                  ,'ConstantDataPointer' ,'CONSTANT_DATA' ,'FUEL_LEVEL_FILTER_SLOW_VALUE')    [cdata.m]    (also in hc12_cdata.m; m4_cdata.m)
  CD_FUEL_PRIMARY_FEEDBACK_FUNCTION = Bobcat.Parameter; CreateAliasCD( CD_FUEL_PRIMARY_FEEDBACK_FUNCTION                    ,uint8(0)                   ,'ConstantDataPointer' ,'CONSTANT_DATA' ,'FUEL_PRIMARY_FEEDBACK_FUNCTION')    [cdata.m]    (also in hc12_cdata.m; m4_cdata.m)
  ... (+246 more)
undefined symbols (not defined in the file or in its folder): sigbuilder_block
undefined symbols (not defined in the file): CD_FUEL_RESOLUTION_TIMER
libraries: simulink x186, MX_Library x107
(part 15 of 48)

== system MX_Gateway/MX_Gtwy_Control/Outputs/Diverter ==
  state_Engine : Inport
  mode_LeftControl : Inport  Port=2
  state_Console : Inport  Port=3
  calc_Vbattery : Inport  Port=4
  state_Vbattery : Inport  Port=5
  state_System : Inport  Port=6
  afbk_Diverter : Inport  Port=7
  ifbk_Diverter : Inport  Port=8
  ain_PrecRef : Inport  Port=9
  din_SecondAuxSw : Inport  Port=10
  CompareToConstant : simulink/Logic and Bit Operations/Compare To Constant  relop=~=  const=NON_FUNCTIONAL  OutDataTypeStr=boolean  ZeroCross=off
  CompareToConstant1 : simulink/Logic and Bit Operations/Compare To Constant  relop===  const=SYS_NORMAL  OutDataTypeStr=boolean  ZeroCross=off
  CompareToConstant2 : simulink/Logic and Bit Operations/Compare To Constant  relop=~=  const=NON_FUNCTIONAL  OutDataTypeStr=boolean  ZeroCross=off
  CompareToConstant3 : simulink/Logic and Bit Operations/Compare To Constant  relop=~=  const=NON_FUNCTIONAL  OutDataTypeStr=boolean  ZeroCross=off
  Constant : Constant  Value=CD_SECOND_AUX_FUNCTION
  Constant1 : Constant  Value=CD_EMEA_CONTROLS_OPTION_A
  Constant2 : Constant  Value=CD_EMEA_CONTROLS_OPTION_C
  Diverter : Subsystem
  Logical Operator : Logic  AllPortsSameDT=off  OutDataTypeStr=boolean
  Logical Operator1 : Logic  Operator=OR  Inputs=3  AllPortsSameDT=off  OutDataTypeStr=boolean
  state_Diverter : Outport  OutputWhenDisabled=reset  InitialOutput=[0]
  hs_Diverter : Outport  Port=2  OutputWhenDisabled=reset  InitialOutput=[0]
  ls_Diverter : Outport  Port=3  OutputWhenDisabled=reset  InitialOutput=[0]
  cmd_Diverter : Outport  Port=4  OutputWhenDisabled=reset  InitialOutput=[0]
  wires:
  state_System/1 -> CompareToConstant1/1
  state_Engine/1 -> Diverter/1(state_Engine)
  state_Console/1 -> Diverter/2(state_Console)
  state_Vbattery/1 -> Diverter/3(state_Vbattery)
  afbk_Diverter/1 -> Diverter/4(afbk_Diverter)
  ifbk_Diverter/1 -> Diverter/5(ifbk_Diverter)
  calc_Vbattery/1 -> Diverter/6(calc_Vbattery)
  Diverter/1(state_Diverter) -> state_Diverter/1
  Diverter/2(hs_Diverter) -> hs_Diverter/1
  Diverter/3(ls_Diverter) -> ls_Diverter/1
  ain_PrecRef/1 -> Diverter/7(ain_PrecRef)
  Constant/1 -> CompareToConstant/1
  Logical Operator/1 -> Diverter/Enable
  CompareToConstant1/1 -> Logical Operator/1
  din_SecondAuxSw/1 -> Diverter/8(din_SecondAuxSw)
  Diverter/4(cmd_Diverter) -> cmd_Diverter/1
  Constant1/1 -> CompareToConstant2/1
  Constant2/1 -> CompareToConstant3/1
  CompareToConstant/1 -> Logical Operator1/1
  Logical Operator1/1 -> Logical Operator/2
  CompareToConstant3/1 -> Logical Operator1/3
  CompareToConstant2/1 -> Logical Operator1/2
  mode_LeftControl/1 -> Diverter/9(mode_LeftControl)

== system MX_Gateway/MX_Gtwy_Control/Outputs/Dump_Valve ==
  aux_Flow : Inport
  state_Engine : Inport  Port=2
  calc_Vbattery : Inport  Port=3
  state_Vbattery : Inport  Port=4
  state_System : Inport  Port=5
  afbk_DumpValve : Inport  Port=6
  ifbk_DumpValve : Inport  Port=7
  ain_PrecRef : Inport  Port=8
  CompareToConstant : simulink/Logic and Bit Operations/Compare To Constant  relop=~=  const=NON_FUNCTIONAL  OutDataTypeStr=boolean  ZeroCross=off
  CompareToConstant1 : simulink/Logic and Bit Operations/Compare To Constant  relop===  const=SYS_NORMAL  OutDataTypeStr=boolean  ZeroCross=off
  Constant : Constant  Value=CD_DUMP_VALVE_FUNCTION
  Dump_Valve : Subsystem
  Logical Operator : Logic  AllPortsSameDT=off  OutDataTypeStr=boolean
  state_DumpValve : Outport  OutputWhenDisabled=reset  InitialOutput=[0]
  hs_DumpValve : Outport  Port=2  OutputWhenDisabled=reset  InitialOutput=[0]
  ls_DumpValve : Outport  Port=3  OutputWhenDisabled=reset  InitialOutput=[0]
  errorCC_DumpValve : Outport  Port=4  OutputWhenDisabled=reset  InitialOutput=[0]
  calc_ifbk_DumpValve : Outport  Port=5  OutputWhenDisabled=reset  InitialOutput=[0]
  wires:
  state_System/1 -> CompareToConstant1/1
  aux_Flow/1 -> Dump_Valve/1(aux_Flow)
  state_Vbattery/1 -> Dump_Valve/3(state_Vbattery)
  afbk_DumpValve/1 -> Dump_Valve/4(afbk_DumpValve)
  ifbk_DumpValve/1 -> Dump_Valve/5(ifbk_DumpValve)
  calc_Vbattery/1 -> Dump_Valve/6(calc_Vbattery)
  Dump_Valve/1(state_DumpValve) -> state_DumpValve/1
  Dump_Valve/2(hs_DumpValve) -> hs_DumpValve/1
  Dump_Valve/3(ls_DumpValve) -> ls_DumpValve/1
  ain_PrecRef/1 -> Dump_Valve/7(ain_PrecRef)
  Constant/1 -> CompareToConstant/1
  CompareToConstant/1 -> Logical Operator/2
  Logical Operator/1 -> Dump_Valve/Enable
  CompareToConstant1/1 -> Logical Operator/1
  state_Engine/1 -> Dump_Valve/2(state_Engine)
  Dump_Valve/4(errorCC_DumpValve) -> errorCC_DumpValve/1
  Dump_Valve/5(calc_ifbk_DumpValve) -> calc_ifbk_DumpValve/1

== system MX_Gateway/MX_Gtwy_Control/Outputs/FuelPri_HVAC ==
  state_Engine : Inport  OutDataTypeStr=uint8
  dfbk_FuelPriOutput : Inport  Port=2
  dfbk_HVACOutput : Inport  Port=3
  din_FuelPriRelay : Inport  Port=4  OutDataTypeStr=boolean
  state_System : Inport  Port=5  OutDataTypeStr=uint8
  error_FuelSec : Inport  Port=6  OutDataTypeStr=uint8
  state_VBattery : Inport  Port=7  OutDataTypeStr=uint8
  flt_ECT : Inport  Port=8
  timer_Resolution : Inport  Port=9  OutDataTypeStr=uint8
  Fuel_Primary_Relay : Subsystem  TreatAsAtomicUnit=on
      settings: RTWSystemCode=Nonreusable function  RTWFcnNameOpts=Use subsystem name
  HVAC_Relay : Subsystem  TreatAsAtomicUnit=on  SystemSampleTime=srate  RTWFileName=mod_Outputs
      settings: RTWSystemCode=Nonreusable function  RTWFileNameOpts=User specified
  dout_FuelPri : Outport
  state_FuelPriOutput : Outport  Port=2
  state_FuelPriRelay : Outport  Port=3
  dout_HVACOutput : Outport  Port=4
  state_HVACOutput : Outport  Port=5
  wires:
  state_Engine/1 -> [state_Engine]  "state_Engine"
  din_FuelPriRelay/1 -> [din_FuelPriRelay]  "din_FuelPriRelay"
  state_VBattery/1 -> [state_VBattery]  "state_VBattery"
  [state_VBattery] -> Fuel_Primary_Relay/7(state_VBattery)  "state_VBattery"
  [state_Engine] -> Fuel_Primary_Relay/4(state_Engine)
  [dfbk_FuelPriOutput] -> Fuel_Primary_Relay/1(dfbk_FuelPriOutput)  "dfbk_FuelPriOutput"
  [din_FuelPriRelay] -> Fuel_Primary_Relay/2(din_FuelPriRelay)  "din_FuelPriRelay"
  dfbk_FuelPriOutput/1 -> [dfbk_FuelPriOutput]  "dfbk_FuelPriOutput"
  [dfbk_HVACOutput] -> HVAC_Relay/2(dfbk_HVACOutput)
  [state_Engine] -> HVAC_Relay/1(state_Engine)
  error_FuelSec/1 -> Fuel_Primary_Relay/3(error_FuelSec)  "error_FuelSec"
  flt_ECT/1 -> Fuel_Primary_Relay/5(flt_ECT)  "flt_ECT"
  timer_Resolution/1 -> Fuel_Primary_Relay/6(timer_Resolution)  "timer_Resolution"
  Fuel_Primary_Relay/1(dout_FuelPri) -> dout_FuelPri/1  "dout_FuelPri"
  Fuel_Primary_Relay/2(state_FuelPriOutput) -> state_FuelPriOutput/1  "state_FuelPriOutput"
  Fuel_Primary_Relay/3(state_FuelPriRelay) -> state_FuelPriRelay/1  "state_FuelPriRelay"
  HVAC_Relay/1(dout_HVACOutput) -> dout_HVACOutput/1
  HVAC_Relay/2(state_HVACOutput) -> state_HVACOutput/1
  state_System/1 -> HVAC_Relay/4(state_System)
  dfbk_HVACOutput/1 -> [dfbk_HVACOutput]
  [state_VBattery] -> HVAC_Relay/3(state_Vbattery)

== system MX_Gateway/MX_Gtwy_Control/Outputs/FuelSec_ECUHold ==
  state_Engine : Inport  OutDataTypeStr=uint8
  state_VBattery : Inport  Port=2  OutDataTypeStr=uint8
  calc_VBattery : Inport  Port=3
  afbk_FuelSec : Inport  Port=4  OutDataTypeStr=uint16
  state_System : Inport  Port=5  OutDataTypeStr=uint8
  din_StarterRelay : Inport  Port=6  OutDataTypeStr=boolean
  state_StarterOutput : Inport  Port=7  OutDataTypeStr=uint8
  state_StarterRelay : Inport  Port=8  OutDataTypeStr=uint8
  Compare To Constant : simulink/Logic and Bit Operations/Compare To Constant  relop=~=  const=NON_FUNCTIONAL  OutDataTypeStr=boolean  ZeroCross=off
  Compare To Constant1 : simulink/Logic and Bit Operations/Compare To Constant  relop=~=  const=OORL  OutDataTypeStr=boolean  ZeroCross=off
  Compare To Constant2 : simulink/Logic and Bit Operations/Compare To Constant  relop=~=  const=NON_FUNCTIONAL  OutDataTypeStr=boolean  ZeroCross=off
  FunctionState : Constant  Value=CD_FUEL_SECONDARY_FEEDBACK_FUNCTION  OutDataTypeStr=uint8
  FunctionState1 : Constant  Value=CD_ECU_FUNCTION  OutDataTypeStr=uint8
  Gain : Gain  Gain=CD_FUEL_SECONDARY_AFBK_GAIN  ParamDataTypeStr=fixdt(0,16,8)  OutDataTypeStr=fixdt(0,16,8)
  Logical Operator : Logic  AllPortsSameDT=off  OutDataTypeStr=boolean  SampleTime=srate
  Logical Operator1 : Logic  Operator=OR  AllPortsSameDT=off  OutDataTypeStr=boolean  SampleTime=srate
  Solenoid : Stateflow chart  TreatAsAtomicUnit=on
  Unit Delay1 : UnitDelay  SampleTime=srate
      settings: AttributesFormatString=<initial=%<X0>>\n<tsample=%<SampleTime>>
  hc_hsd : MX_Library/hc_hsd  STB_DB_CNT=CD_FUEL_SECONDARY_DEBOUNCE_SHORT_TO_BATTERY  STG_DB_CNT=CD_FUEL_SECONDARY_DEBOUNCE_SHORT_TO_GROUND  OPEN_DB_CNT=CD_FUEL_SECONDARY_DEBOUNCE_OPEN_CIRCUIT
  duty_FuelSec : Outport
  state_FuelSec : Outport  Port=2  OutDataTypeStr=uint8
  error_FuelSec : Outport  Port=3  OutDataTypeStr=uint8
  state_ECUHold : Outport  Port=4  OutDataTypeStr=uint8
  wires:
  Solenoid/2(state_FuelSec) -> state_FuelSec/1  "state_FuelSec"
  state_System/1 -> Solenoid/3(state_System)  "state_System"
  state_Engine/1 -> [state_Engine]  "state_Engine"
  state_VBattery/1 -> [state_VBattery]  "state_VBattery"
  Logical Operator/1 -> hc_hsd/Enable  "EnableDetection"
  Compare To Constant1/1 -> Logical Operator/2
  Logical Operator1/1 -> Logical Operator/1
  [state_VBattery] -> Compare To Constant1/1  "state_VBattery"
  Gain/1 -> hc_hsd/2  "afbkGain_FuelSec"
  Unit Delay1/1 -> Solenoid/2(error_FuelSec)  "error_FuelSec"
  Solenoid/1(duty_FuelSec) -> duty_FuelSec/1, hc_hsd/1  "duty_FuelSec"
  hc_hsd/1 -> error_FuelSec/1, Unit Delay1/1  "error_FuelSec"
  afbk_FuelSec/1 -> Gain/1  "afbk_FuelSec"
  calc_VBattery/1 -> hc_hsd/3  "calc_VBattery"
  [state_Engine] -> Solenoid/1(state_Engine)  "state_Engine"
  Solenoid/3(state_ECUHold) -> state_ECUHold/1  "state_ECUHold"
  FunctionState1/1 -> Compare To Constant2/1
  Compare To Constant2/1 -> Logical Operator1/2
  Compare To Constant/1 -> Logical Operator1/1
  FunctionState/1 -> Compare To Constant/1
  state_StarterOutput/1 -> Solenoid/4(state_StarterOutput)  "state_StarterOutput"
  state_StarterRelay/1 -> Solenoid/5(state_StarterRelay)  "state_StarterRelay"
  din_StarterRelay/1 -> Solenoid/6(din_StarterRelay)  "din_StarterRelay"

== system MX_Gateway/MX_Gtwy_Control/Outputs/Fuel_Resolution_Timer ==
  dout_Primary : Inport
  Timer : Stateflow chart  TreatAsAtomicUnit=on
  state_Timer : Outport
  wires:
  Timer/1(state_Timer) -> state_Timer/1  "state_Timer"
  dout_Primary/1 -> Timer/1(dout_Primary)  "dout_Primary"

== system MX_Gateway/MX_Gtwy_Control/Outputs/GlowPlug ==
  state_Engine : Inport
  state_VBattery : Inport  Port=2
  flt_HOT : Inport  Port=3
  flt_ECT : Inport  Port=4
  dfbk_GlowPlugOutput : Inport  Port=5
  din_GlowPlugRelay : Inport  Port=6
  state_System : Inport  Port=7
  ecu_GlowPlug : Inport  Port=8
  ecu_ECT : Inport  Port=9
  state_ecuECT : Inport  Port=10
  Compare To Constant : simulink/Logic and Bit Operations/Compare To Constant  relop=~=  const=NON_FUNCTIONAL  OutDataTypeStr=boolean  ZeroCross=off
  Compare To Constant1 : simulink/Logic and Bit Operations/Compare To Constant  relop=~=  const=OORL  OutDataTypeStr=boolean  ZeroCross=off
  Compare To Constant2 : simulink/Logic and Bit Operations/Compare To Constant  relop=~=  const=NON_FUNCTIONAL  OutDataTypeStr=boolean  ZeroCross=off
  ECU_Glow_Time : Lookup  InputValues=MXGTW_ECU_GLOW_TEMP  Table=MXGTW_ECU_GLOW_TIME  LookUpMeth=Interpolation-Use End Values  OutDataTypeStr=uint16  SaturateOnIntegerOverflow=on
      settings: AttributesFormatString=input = %<InputValues>\noutput = %<OutputValues>
  FunctionState : Constant  Value=CD_GLOW_PLUG_PREHEAT_FEEDBACK_FUNCTION  OutDataTypeStr=uint8
  FunctionState1 : Constant  Value=CD_HOT_FUNCTION  OutDataTypeStr=uint8
  GlowPlug : Stateflow chart  TreatAsAtomicUnit=on
  Logical Operator : Logic  AllPortsSameDT=off  OutDataTypeStr=boolean
  Logical Operator1 : Logic  AllPortsSameDT=off  OutDataTypeStr=boolean
  Relational Operator : RelationalOperator  Operator=<  OutDataTypeStr=boolean  ZeroCross=off  RndMeth=Nearest
  Switch : Switch  Criteria=u2 > Threshold  ZeroCross=off
  Unit Delay : UnitDelay  InitialCondition=8  SampleTime=-1
      settings: AttributesFormatString=<initial=%<X0>>\n<tsample=%<SampleTime>>
  Unit Delay1 : UnitDelay  InitialCondition=8  SampleTime=-1
      settings: AttributesFormatString=<initial=%<X0>>\n<tsample=%<SampleTime>>
  relay_oc : MX_Library/relay_oc  ERROR_ON_DB_CNT=CD_GLOW_PLUG_DEBOUNCE_ERROR_ON  ERROR_OFF_DB_CNT=CD_GLOW_PLUG_DEBOUNCE_ERROR_OFF  NORMAL_DB_CNT=CD_GLOW_PLUG_DEBOUNCE_NORMAL
  dout_GlowPlug : Outport
  state_GlowPlugOutput : Outport  Port=2
  state_GlowPlugRelay : Outport  Port=3
  count_GlowPlug : Outport  Port=4
  wires:
  [state_VBattery] -> GlowPlug/2(state_VBattery)  "state_VBattery"
  state_System/1 -> GlowPlug/3(state_System)  "state_System"
  GlowPlug/2(count_GlowPlug) -> count_GlowPlug/1  "count_GlowPlug"
  state_VBattery/1 -> [state_VBattery]  "state_VBattery"
  Logical Operator1/1 -> relay_oc/Enable  "EnableDetection"
  Compare To Constant1/1 -> Logical Operator1/2
  Compare To Constant/1 -> Logical Operator1/1
  [state_VBattery] -> Compare To Constant1/1
  FunctionState/1 -> Compare To Constant/1
  Unit Delay1/1 -> GlowPlug/9(state_GlowPlugRelay)  "state_GlowPlugRelay"
  Unit Delay/1 -> GlowPlug/8(state_GlowPlugOutput)  "state_GlowPlugOutput"
  GlowPlug/1(dout_GlowPlug) -> dout_GlowPlug/1, relay_oc/1  "dout_GlowPlug"
  state_Engine/1 -> GlowPlug/1(state_Engine)  "state_Engine"
  din_GlowPlugRelay/1 -> relay_oc/3  "din_GlowPlugRelay"
  dfbk_GlowPlugOutput/1 -> relay_oc/2  "dfbk_GlowPlugOutput"
  relay_oc/2 -> Unit Delay1/1, state_GlowPlugRelay/1  "state_GlowPlugRelay"
  relay_oc/1 -> Unit Delay/1, state_GlowPlugOutput/1  "state_GlowPlugOutput"
  FunctionState1/1 -> Compare To Constant2/1
  Compare To Constant2/1 -> Logical Operator/1
  Relational Operator/1 -> Logical Operator/2
  Logical Operator/1 -> Switch/2
  Switch/1 -> GlowPlug/4(MachineTemp)  "temp_Lowest"
  flt_HOT/1 -> Relational Operator/1, Switch/1  "flt_HOT"
  flt_ECT/1 -> Relational Operator/2, Switch/3  "flt_ECT"
  ECU_Glow_Time/1 -> GlowPlug/5(time_GlowPlug)  "time_GlowPlug"
  ecu_GlowPlug/1 -> GlowPlug/6(ecu_GlowPlug)
  ecu_ECT/1 -> ECU_Glow_Time/1
  state_ecuECT/1 -> GlowPlug/7(state_ecuECT)

== system MX_Gateway/MX_Gtwy_Control/Outputs/Hyd_Bypass ==
  state_Engine : Inport
  state_Console : Inport  Port=2
  calc_Vbattery : Inport  Port=3
  state_Vbattery : Inport  Port=4
  state_System : Inport  Port=5
  afbk_HydBypass : Inport  Port=6
  ifbk_HydBypass : Inport  Port=7
  ain_PrecRef : Inport  Port=8
  CompareToConstant : simulink/Logic and Bit Operations/Compare To Constant  relop=~=  const=NON_FUNCTIONAL  OutDataTypeStr=boolean  ZeroCross=off
  CompareToConstant1 : simulink/Logic and Bit Operations/Compare To Constant  relop===  const=SYS_NORMAL  OutDataTypeStr=boolean  ZeroCross=off
  Constant : Constant  Value=CD_HYD_BYPASS_FUNCTION
  Hyd_Bypass : Subsystem
  Logical Operator : Logic  AllPortsSameDT=off  OutDataTypeStr=boolean
  state_HydBypass : Outport  OutputWhenDisabled=reset  InitialOutput=[0]
  hs_HydBypass : Outport  Port=2  OutputWhenDisabled=reset  InitialOutput=[0]
  ls_HydBypass : Outport  Port=3  OutputWhenDisabled=reset  InitialOutput=[0]
  wires:
  state_System/1 -> CompareToConstant1/1
  state_Engine/1 -> Hyd_Bypass/1(state_Engine)
  state_Console/1 -> Hyd_Bypass/2(state_Console)
  state_Vbattery/1 -> Hyd_Bypass/3(state_Vbattery)
  afbk_HydBypass/1 -> Hyd_Bypass/4(afbk_HydBypass)
  ifbk_HydBypass/1 -> Hyd_Bypass/5(ifbk_HydBypass)
  calc_Vbattery/1 -> Hyd_Bypass/6(calc_Vbattery)
  Hyd_Bypass/1(state_HydBypass) -> state_HydBypass/1
  Hyd_Bypass/2(hs_HydBypass) -> hs_HydBypass/1
  Hyd_Bypass/3(ls_HydBypass) -> ls_HydBypass/1
  ain_PrecRef/1 -> Hyd_Bypass/7(ain_PrecRef)
  Constant/1 -> CompareToConstant/1
  CompareToConstant/1 -> Logical Operator/2
  Logical Operator/1 -> Hyd_Bypass/Enable
  CompareToConstant1/1 -> Logical Operator/1

== system MX_Gateway/MX_Gtwy_Control/Outputs/Hyd_Enable ==
  note: Possible status for state_Engine
      | #define ENGINE_INITIALIZE   0
      | #define ENGINE_NOT_RUNNING  1
      | #define ENGINE_CRANKING     2
      | #define ENGINE_RUNNING      3
      | #define ENGINE_OFF          4
  note: Possible controller system_states
      |   SYS_INIT,
      |   SYS_NORMAL,
      |   SYS_DV,
      |   SYS_LIMITED,
      |   SYS_POWER_DOWN,
      |   SYS_PHONE_LIMITED
  state_Engine : Inport
  state_Console : Inport  Port=2
  calc_Vbattery : Inport  Port=3
  state_Vbattery : Inport  Port=4
  state_System : Inport  Port=5
  afbk_HydEnable : Inport  Port=6
  ifbk_HydEnable : Inport  Port=7
  ain_PrecRef : Inport  Port=8
  In1 : Inport  Port=9
  CompareToConstant1 : simulink/Logic and Bit Operations/Compare To Constant  relop===  const=SYS_NORMAL  OutDataTypeStr=boolean  ZeroCross=off
  Hyd_Enable : Subsystem
  state_HydEnable : Outport  OutputWhenDisabled=reset  InitialOutput=[0]
  hs_HydEnable : Outport  Port=2  OutputWhenDisabled=reset  InitialOutput=[0]
  ls_HydEnable : Outport  Port=3  OutputWhenDisabled=reset  InitialOutput=[0]
  wires:
  state_System/1 -> CompareToConstant1/1
  CompareToConstant1/1 -> Hyd_Enable/Enable
  state_Engine/1 -> Hyd_Enable/1(state_Engine)
  state_Console/1 -> Hyd_Enable/2(state_Console)
  state_Vbattery/1 -> Hyd_Enable/3(state_Vbattery)
  afbk_HydEnable/1 -> Hyd_Enable/4(afbk_HydEnable)
  ifbk_HydEnable/1 -> Hyd_Enable/5(ifbk_HydEnable)
  calc_Vbattery/1 -> Hyd_Enable/6(calc_Vbattery)
  Hyd_Enable/1(state_HydEnable) -> state_HydEnable/1
  Hyd_Enable/2(hs_HydEnable) -> hs_HydEnable/1
  Hyd_Enable/3(ls_HydEnable) -> ls_HydEnable/1
  ain_PrecRef/1 -> Hyd_Enable/7(ain_PrecRef)
  In1/1 -> Hyd_Enable/8(state_Inverter)

== system MX_Gateway/MX_Gtwy_Control/Outputs/Hydraulic_Exchange ==
  state_Console : Inport
  state_Vbattery : Inport  Port=2
  dfbk_HydExchange : Inport  Port=3
  Compare To Constant : simulink/Logic and Bit Operations/Compare To Constant  relop=~=  const=NON_FUNCTIONAL  OutDataTypeStr=boolean  ZeroCross=off
  Compare To Constant1 : simulink/Logic and Bit Operations/Compare To Constant  relop=~=  const=OORL  OutDataTypeStr=boolean  ZeroCross=off
  FunctionState : Constant  Value=CD_HYD_EXCHANGE_FEEDBACK_FUNCTION  OutDataTypeStr=uint8
  Hydraulic_Xchange : Stateflow chart  TreatAsAtomicUnit=on
  Logical Operator : Logic  AllPortsSameDT=off  OutDataTypeStr=boolean
  Unit Delay1 : UnitDelay  SampleTime=-1
      settings: AttributesFormatString=<initial=%<X0>>\n<tsample=%<SampleTime>>
  dout_chk : MX_Library/dout_chk  ERR_ON_DB_CNT=CD_HYD_EXCHANGE_DEBOUNCE_ERROR_ON  ERR_OFF_DB_CNT=CD_HYD_EXCHANGE_DEBOUNCE_ERROR_OFF
  state_HydExchange : Outport  OutDataTypeStr=uint8
  dout_HydExchange : Outport  Port=2  OutputWhenDisabled=reset  InitialOutput=[0]
  wires:
  state_Console/1 -> Hydraulic_Xchange/1(state_Console)
  state_Vbattery/1 -> [state_Vbattery]
  dout_chk/1 -> state_HydExchange/1, [state_Output]
  dfbk_HydExchange/1 -> dout_chk/2
  Compare To Constant1/1 -> Logical Operator/2  "EnableFeedback"
  Logical Operator/1 -> dout_chk/Enable
  FunctionState/1 -> Compare To Constant/1
  Compare To Constant/1 -> Logical Operator/1
  Hydraulic_Xchange/1(duty_HydExchange) -> dout_HydExchange/1, dout_chk/1
  Unit Delay1/1 -> Hydraulic_Xchange/2(state_Output)
  [state_Output] -> Unit Delay1/1
  [state_Vbattery] -> Compare To Constant1/1
